simulink model "TosAeb"  (R2021a)
settings: Solver=FixedStepDiscrete  StartTime=0.0  StopTime=inf  FixedStep=0.01  RelTol=1e-3
undefined symbols (not defined in the file or in its folder): ACCModSts, AccSts1, AEB_ObjectMovingOrientation, AEB_ObjectMovingState, CN_BTCActive, CN_CurrentGearR, CN_CurrentGearR_DHT, CN_PTCActive, CN_VDCActive, CN_VSC_EspOff, CN_VSC_FCW_AEB_Error, CN_VSC_FCW_AEB_NoDispay, CN_VSC_FCW_AEB_PerfmDgdtn, CN_VSC_FCW_AEB_TempUnAvlbl, CN_VSC_VehStandstill, CNaccelAvoidMax, CNaccelAvoidMin, CNbrakePedalPress, CNcomfortStrLeftAccelMax, CNcomfortStrLeftAccelMin, CNcomfortStrRightAccelMax, CNcomfortStrRightAccelMin, CNDrvDoorOpen, CNDrvModeEco, CNDrvModeNorm, CNDrvModeOther, CNDrvModeSport, CNDrvSBRreminder, CNNull, CNobjInvalidID, CNPassDoorOpen, CNPassSBRreminder, CNPEBSAccelNegInf, CNPEBSAccelNull, CNPEBSTimeInf, CNPEBSTimeNull, CNSTOPMAX, CNSTOPNULL, CNsubjWidth, CNSwtOFF, CNSwtON, CNTTCMAX, CNTTCNULL, CNVehConfigAEBCycNone, CNVehConfigAEBNone, CNVehConfigAEBPedNone, CNVehConfigFCWNone, CNVehConfigJANone, CollisionSeverity, FALSE, FCWSnvty, JACycObjCalcInfoDefaultParams, JAPedObjCalcInfoDefaultParams, JAVehObjCalcInfoDefaultParams, latCycObjCalcInfoDefaultParams, latPedObjCalcInfoDefaultParams, longObjCalcInfoDefaultParams, ModSts, movingStatus, ObjectType ... (+1112)
libraries: simulink x3031, AEBSFunctionLib x50, Ta_Library x33, AEBSStateMachineLib x23, Mylibrary x12, simulink_extras x7, ObjSelect_lib x3, dspstat3 x2
(part 44 of 102)

== system TosAeb/FcwAebJa_Step_sys/ALG_AEBSystem/ALG_AEBController/AEB_FuncCondCalcApp/PedFunctionCondCalc/pedPrefillSuppAborCond/pedPrefillSuppCondCalc/pedPrefillObjRelActiveCondCalc ==
  ObjectID : Inport
  vxAbsObj : Inport  Port=2
  ObjFusQuality : Inport  Port=3
  dy : Inport  Port=4
  vy : Inport  Port=5
  Abs : Abs
  Compare To Constant : simulink/Logic and Bit Operations/Compare To Constant  relop=>  const=CNNull  OutDataTypeStr=boolean
  Compare To Constant1 : simulink/Logic and Bit Operations/Compare To Constant  relop=>  const=P_AEB_Func_pedPrefillVxAbsObjSuppThres  OutDataTypeStr=boolean
  Compare To Constant11 : simulink/Logic and Bit Operations/Compare To Constant  relop===  const=FALSE  OutDataTypeStr=boolean
  Compare To Constant2 : simulink/Logic and Bit Operations/Compare To Constant  relop=>  const=CNNull  OutDataTypeStr=boolean
  Compare To Constant3 : simulink/Logic and Bit Operations/Compare To Constant  relop=<  const=CNNull  OutDataTypeStr=boolean
  Compare To Constant4 : simulink/Logic and Bit Operations/Compare To Constant  relop===  const=CNobjInvalidID  OutDataTypeStr=boolean
  Compare To Constant5 : simulink/Logic and Bit Operations/Compare To Constant  relop=<  const=CNNull  OutDataTypeStr=boolean
  Logical Operator : Logic  Operator=OR  Inputs=3  AllPortsSameDT=off  OutDataTypeStr=boolean
  Logical Operator1 : Logic  AllPortsSameDT=off  OutDataTypeStr=boolean
  Logical Operator2 : Logic  AllPortsSameDT=off  OutDataTypeStr=boolean
  Logical Operator3 : Logic  Operator=OR  AllPortsSameDT=off  OutDataTypeStr=boolean
  Terminator : Terminator
  pedPrefillObjRelSuppCondFulfilled : Outport
  wires:
  Logical Operator2/1 -> Logical Operator3/2
  Logical Operator1/1 -> Logical Operator3/1
  Compare To Constant5/1 -> Logical Operator2/2
  Compare To Constant3/1 -> Logical Operator2/1
  Compare To Constant2/1 -> Logical Operator1/2
  Compare To Constant/1 -> Logical Operator1/1
  vy/1 -> Compare To Constant2/1, Compare To Constant5/1
  dy/1 -> Compare To Constant/1, Compare To Constant3/1
  Compare To Constant11/1 -> Logical Operator/3  "ObjFusQuality"
  ObjFusQuality/1 -> Compare To Constant11/1
  Logical Operator/1 -> pedPrefillObjRelSuppCondFulfilled/1
  Compare To Constant4/1 -> Logical Operator/1
  Compare To Constant1/1 -> Logical Operator/2
  Abs/1 -> Compare To Constant1/1
  vxAbsObj/1 -> Abs/1
  ObjectID/1 -> Compare To Constant4/1
  Logical Operator3/1 -> Terminator/1

== system TosAeb/FcwAebJa_Step_sys/ALG_AEBSystem/ALG_AEBController/AEB_FuncCondCalcApp/PedFunctionCondCalc/pedPrefillSuppAborCond/pedPrefillSuppCondCalc/pedPrefillSituationRelActiveCondCalc ==
  latCollisionSeverity : Inport
  subjCurvTraj : Inport  Port=2
  subjLatAccel : Inport  Port=3
  colliisonRiskProb : Inport  Port=4
  ObjectTypeCameraConfirmed : Inport  Port=5
  dy : Inport  Port=6
  vy : Inport  Port=7
  dxFrontBumper : Inport  Port=8
  longDist4Brk : Inport  Port=9
  distSecure : Inport  Port=10
  subVel : Inport  Port=11
  objLatVyCond4PrefillCalc : Subsystem
  objLongDistCond4PrefillCalc : Subsystem
  pedPrefillSituationRelSuppCond : Subsystem
  pedPrefillSituationRelSuppCondFulfilled : Outport
  wires:
  objLongDistCond4PrefillCalc/1(ObjLongDistCond4Prefill) -> pedPrefillSituationRelSuppCond/7(objLatVyCond4Prefill)
  objLatVyCond4PrefillCalc/1(ObjLatVyCond4Prefill) -> pedPrefillSituationRelSuppCond/6(objLongDistCond4Prefill)
  distSecure/1 -> objLongDistCond4PrefillCalc/3(distSecure)
  subVel/1 -> objLongDistCond4PrefillCalc/4(subVel)
  longDist4Brk/1 -> objLongDistCond4PrefillCalc/2(longDist4Brk)
  pedPrefillSituationRelSuppCond/1(pedPrefillSituationRelActiveCondFulfilled) -> pedPrefillSituationRelSuppCondFulfilled/1
  ObjectTypeCameraConfirmed/1 -> pedPrefillSituationRelSuppCond/5(ObjectTypeCameraConfirmed), objLatVyCond4PrefillCalc/1(objObjectTypeCameraConfirmed)
  colliisonRiskProb/1 -> pedPrefillSituationRelSuppCond/4(colliisonRiskProb)
  subjLatAccel/1 -> pedPrefillSituationRelSuppCond/3(subjLatAccel)
  subjCurvTraj/1 -> pedPrefillSituationRelSuppCond/2(subjCurvTraj)
  latCollisionSeverity/1 -> pedPrefillSituationRelSuppCond/1(latCollisionSeverity)
  dy/1 -> objLatVyCond4PrefillCalc/2(dy)
  vy/1 -> objLatVyCond4PrefillCalc/3(vy)
  dxFrontBumper/1 -> objLongDistCond4PrefillCalc/1(dxFrontBumper)

== system TosAeb/FcwAebJa_Step_sys/ALG_AEBSystem/ALG_AEBController/AEB_FuncCondCalcApp/PedFunctionCondCalc/pedPrefillSuppAborCond/pedPrefillSuppCondCalc/pedPrefillSuppCondFinalJudge ==
  pedPrefillObjRelSuppCondFulfilled : Inport
  pedPrefillVehRelSuppCondFulfilled : Inport  Port=2
  pedPrefillSituationRelSuppCondFulfilled : Inport  Port=3
  Compare To Constant : simulink/Logic and Bit Operations/Compare To Constant  relop===  const=TRUE  OutDataTypeStr=boolean
  Compare To Constant1 : simulink/Logic and Bit Operations/Compare To Constant  relop===  const=TRUE  OutDataTypeStr=boolean
  Compare To Constant2 : simulink/Logic and Bit Operations/Compare To Constant  relop===  const=TRUE  OutDataTypeStr=boolean
  Logical Operator : Logic  Operator=OR  Inputs=3  AllPortsSameDT=off  OutDataTypeStr=boolean
  pedPrefillSuppCondFulfilled : Outport
  wires:
  Compare To Constant2/1 -> Logical Operator/3
  Compare To Constant1/1 -> Logical Operator/2
  Compare To Constant/1 -> Logical Operator/1
  pedPrefillVehRelSuppCondFulfilled/1 -> Compare To Constant1/1
  pedPrefillObjRelSuppCondFulfilled/1 -> Compare To Constant/1
  pedPrefillSituationRelSuppCondFulfilled/1 -> Compare To Constant2/1
  Logical Operator/1 -> pedPrefillSuppCondFulfilled/1

== system TosAeb/FcwAebJa_Step_sys/ALG_AEBSystem/ALG_AEBController/AEB_FuncCondCalcApp/PedFunctionCondCalc/pedPrefillSuppAborCond/pedPrefillSuppCondCalc/pedPrefillVehRelActiveCondCalc ==
  subjVel : Inport
  VehConfigIbooster : Inport  Port=2
  ESP_MasterCylindBrakePressValid : Inport  Port=3
  ESP_MasterCylindBrakePress : Inport  Port=4
  IB_BrkPedalAppld : Inport  Port=5
  SteerWheelAngle : Inport  Port=6
  SteerWheelSpd : Inport  Port=7
  AccrPedlPosnDiagc : Inport  Port=8
  vehStateCalcpedPrefillSuppFlg : Inport  Port=9
  Abs : Abs
  Abs1 : Abs
  AccReleventSuppCondStateDelayAbortion : AEBSFunctionLib/StateDelayAbortion  SystemSampleTime=-1  VariantActivationTime=update diagram  Latency=0  AutoFrameSizeCalculation=off
  Compare To Constant : simulink/Logic and Bit Operations/Compare To Constant  relop=>  const=P_AEB_Func_pedPrefillStrWheelAngleSupThres  OutDataTypeStr=boolean
  Compare To Constant1 : simulink/Logic and Bit Operations/Compare To Constant  relop=>  const=P_AEB_Func_pedPrefillGasPedalSupThres  OutDataTypeStr=boolean
  Compare To Constant2 : simulink/Logic and Bit Operations/Compare To Constant  relop=>  const=P_AEB_Func_pedPrefillStrWheelAngleSpdSupThres  OutDataTypeStr=boolean
  Compare To Constant7 : simulink/Logic and Bit Operations/Compare To Constant  relop===  const=TRUE  OutDataTypeStr=boolean
  Compare To Constant8 : simulink/Logic and Bit Operations/Compare To Constant  relop=>  const=P_AEB_Func_pedPrefillSubjVelMax  OutDataTypeStr=boolean
  Compare To Constant9 : simulink/Logic and Bit Operations/Compare To Constant  relop=<  const=P_AEB_Func_pedPrefillSubjVelMin  OutDataTypeStr=boolean
  Constant : Constant  Value=P_AEB_Func_pedPrefillStrCondAborDelayTime
  Constant1 : Constant  Value=0.02
  Constant2 : Constant  Value=P_AEB_Func_pedPrefillAccrCondAborDelayTime
  Constant3 : Constant  Value=0.02
  Logical Operator : Logic  Operator=OR  AllPortsSameDT=off  OutDataTypeStr=boolean
  Logical Operator4 : Logic  Operator=OR  AllPortsSameDT=off  OutDataTypeStr=boolean
  Logical Operator5 : Logic  Operator=OR  Inputs=5  AllPortsSameDT=off  OutDataTypeStr=boolean
  StrReleventSuppCondDelayAbortion : AEBSFunctionLib/StateDelayAbortion  SystemSampleTime=-1  VariantActivationTime=update diagram  Latency=0  AutoFrameSizeCalculation=off
  brakeReleventSuppCond : Subsystem
  pedPrefillVehRelSuppCondFulfilled : Outport
  wires:
  VehConfigIbooster/1 -> brakeReleventSuppCond/1(VehConfigIbooster)
  IB_BrkPedalAppld/1 -> brakeReleventSuppCond/4(IB_BrkPedalAppld)
  ESP_MasterCylindBrakePress/1 -> brakeReleventSuppCond/3(ESP_MasterCylindBrakePress)
  ESP_MasterCylindBrakePressValid/1 -> brakeReleventSuppCond/2(ESP_MasterCylindBrakePressValid)
  Constant2/1 -> AccReleventSuppCondStateDelayAbortion/2
  Constant3/1 -> AccReleventSuppCondStateDelayAbortion/3
  Compare To Constant1/1 -> AccReleventSuppCondStateDelayAbortion/1
  Constant1/1 -> StrReleventSuppCondDelayAbortion/3
  Constant/1 -> StrReleventSuppCondDelayAbortion/2
  StrReleventSuppCondDelayAbortion/1 -> Logical Operator5/3  "strReleventSuppCondFulfilled"
  Logical Operator4/1 -> StrReleventSuppCondDelayAbortion/1
  AccReleventSuppCondStateDelayAbortion/1 -> Logical Operator5/4  "accReleventSuppCondFulfilled"
  Abs1/1 -> Compare To Constant2/1
  vehStateCalcpedPrefillSuppFlg/1 -> Compare To Constant7/1
  Compare To Constant8/1 -> Logical Operator/1
  Compare To Constant2/1 -> Logical Operator4/2
  SteerWheelSpd/1 -> Abs1/1
  Compare To Constant/1 -> Logical Operator4/1
  Abs/1 -> Compare To Constant/1
  SteerWheelAngle/1 -> Abs/1
  brakeReleventSuppCond/1(brakeReleventSuppCond) -> Logical Operator5/2  "BrkReleventSuppCondFulfilled"
  Compare To Constant9/1 -> Logical Operator/2
  AccrPedlPosnDiagc/1 -> Compare To Constant1/1
  Compare To Constant7/1 -> Logical Operator5/5  "vehStateCalcPedPrefillSuppCondFulfilled"
  Logical Operator/1 -> Logical Operator5/1  "subjVelReleventSuppCondFulfilled"
  subjVel/1 -> Compare To Constant9/1, Compare To Constant8/1
  Logical Operator5/1 -> pedPrefillVehRelSuppCondFulfilled/1  "pedPrefillVehRelSuppCondFulfilled"

== system TosAeb/FcwAebJa_Step_sys/ALG_AEBSystem/ALG_AEBController/AEB_FuncImpManagementApp/FuncImpArbCalc/BrkFuncImpArbCalc/BrkFuncImpAborCondCalc/AEBfullFuncImpAborCondCalc ==
  pedAEBfullModStsArb : Inport
  cycAEBfullModStsArb : Inport  Port=2
  EBAModStsArb : Inport  Port=3
  JA_PTW_AEBfullModStsArb : Inport  Port=4
  JA_cycAEBfullModStsArb : Inport  Port=5
  JA_pedAEBfullModStsArb : Inport  Port=6
  Compare To Constant{3..5,23..25} (6 alike) : simulink/Logic and Bit Operations/Compare To Constant  relop===  const=ModSts.ActiveState  OutDataTypeStr=boolean
  Logical Operator4 : Logic  Operator=OR  Inputs=6  AllPortsSameDT=off  OutDataTypeStr=boolean
  AEBfullFuncImpAborCond : Outport
  wires:
  Compare To Constant3/1 -> Logical Operator4/4
  Compare To Constant4/1 -> Logical Operator4/5
  Compare To Constant5/1 -> Logical Operator4/6
  JA_pedAEBfullModStsArb/1 -> Compare To Constant5/1
  JA_cycAEBfullModStsArb/1 -> Compare To Constant4/1
  JA_PTW_AEBfullModStsArb/1 -> Compare To Constant3/1
  EBAModStsArb/1 -> Compare To Constant25/1
  Compare To Constant24/1 -> Logical Operator4/2
  Compare To Constant23/1 -> Logical Operator4/1
  cycAEBfullModStsArb/1 -> Compare To Constant24/1
  pedAEBfullModStsArb/1 -> Compare To Constant23/1
  Logical Operator4/1 -> AEBfullFuncImpAborCond/1
  Compare To Constant25/1 -> Logical Operator4/3

== system TosAeb/FcwAebJa_Step_sys/ALG_AEBSystem/ALG_AEBController/AEB_FuncImpManagementApp/FuncImpArbCalc/BrkFuncImpArbCalc/BrkFuncImpAborCondCalc/AEBpartFuncImpAborCondCalc ==
  AEBfullModStsArb : Inport
  pedAEBfullModStsArb : Inport  Port=2
  cycAEBpartModStsArb : Inport  Port=3
  cycAEBfullModStsArb : Inport  Port=4
  JA_vehAEBfullModStsArb : Inport  Port=5
  EBAModStsArb : Inport  Port=6
  JA_PTW_AEBfullModStsArb : Inport  Port=7
  JA_cycAEBfullModStsArb : Inport  Port=8
  JA_pedAEBfullModStsArb : Inport  Port=9
  Compare To Constant{1..3,7..12} (9 alike) : simulink/Logic and Bit Operations/Compare To Constant  relop===  const=ModSts.ActiveState  OutDataTypeStr=boolean
  Logical Operator1 : Logic  Operator=OR  Inputs=9  AllPortsSameDT=off  OutDataTypeStr=boolean
  AEBpartFuncImpAborCond : Outport
  wires:
  Compare To Constant3/1 -> Logical Operator1/9
  Compare To Constant2/1 -> Logical Operator1/8
  Compare To Constant1/1 -> Logical Operator1/7
  JA_pedAEBfullModStsArb/1 -> Compare To Constant3/1
  JA_cycAEBfullModStsArb/1 -> Compare To Constant2/1
  JA_PTW_AEBfullModStsArb/1 -> Compare To Constant1/1
  Logical Operator1/1 -> AEBpartFuncImpAborCond/1
  Compare To Constant9/1 -> Logical Operator1/3
  Compare To Constant11/1 -> Logical Operator1/5
  pedAEBfullModStsArb/1 -> Compare To Constant8/1
  Compare To Constant10/1 -> Logical Operator1/4
  Compare To Constant7/1 -> Logical Operator1/1
  Compare To Constant8/1 -> Logical Operator1/2
  EBAModStsArb/1 -> Compare To Constant12/1
  AEBfullModStsArb/1 -> Compare To Constant7/1
  cycAEBpartModStsArb/1 -> Compare To Constant9/1
  cycAEBfullModStsArb/1 -> Compare To Constant10/1
  JA_vehAEBfullModStsArb/1 -> Compare To Constant11/1
  Compare To Constant12/1 -> Logical Operator1/6

== system TosAeb/FcwAebJa_Step_sys/ALG_AEBSystem/ALG_AEBController/AEB_FuncImpManagementApp/FuncImpArbCalc/BrkFuncImpArbCalc/BrkFuncImpAborCondCalc/EBAFuncImpAborCondCalc ==
  Constant : Constant  Value=FALSE
  EBAFuncImpAborCond : Outport
  wires:
  Constant/1 -> EBAFuncImpAborCond/1

== system TosAeb/FcwAebJa_Step_sys/ALG_AEBSystem/ALG_AEBController/AEB_FuncImpManagementApp/FuncImpArbCalc/BrkFuncImpArbCalc/BrkFuncImpAborCondCalc/FCTBFuncImpAborCondCalc ==
  AEBpartModStsArb : Inport
  AEBfullModStsArb : Inport  Port=2
  EBAModStsArb : Inport  Port=3
  pedAEBfullModStsArb : Inport  Port=4
  cycAEBfullModStsArb : Inport  Port=5
  cycAEBpartModStsArb : Inport  Port=6
  JA_vehAEBfullModStsArb : Inport  Port=7
  JA_PTW_AEBModStsArb : Inport  Port=8
  JA_cycAEBModStsArb : Inport  Port=9
  JA_pedAEBModStsArb : Inport  Port=10
  Compare To Constant{,1..9} (10 alike) : simulink/Logic and Bit Operations/Compare To Constant  relop===  const=ModSts.ActiveState  OutDataTypeStr=boolean
  Logical Operator : Logic  Operator=OR  Inputs=10  AllPortsSameDT=off  OutDataTypeStr=boolean
  FCTBFuncImpAborCond : Outport
  wires:
  JA_pedAEBModStsArb/1 -> Compare To Constant9/1
  Compare To Constant9/1 -> Logical Operator/10
  JA_cycAEBModStsArb/1 -> Compare To Constant8/1
  Compare To Constant8/1 -> Logical Operator/9
  Compare To Constant7/1 -> Logical Operator/8
  JA_PTW_AEBModStsArb/1 -> Compare To Constant7/1
  Compare To Constant2/1 -> Logical Operator/3
  Compare To Constant5/1 -> Logical Operator/6
  Compare To Constant/1 -> Logical Operator/1
  Compare To Constant4/1 -> Logical Operator/5
  EBAModStsArb/1 -> Compare To Constant2/1
  Compare To Constant6/1 -> Logical Operator/7
  Compare To Constant3/1 -> Logical Operator/4
  Logical Operator/1 -> FCTBFuncImpAborCond/1
  Compare To Constant1/1 -> Logical Operator/2
  pedAEBfullModStsArb/1 -> Compare To Constant3/1
  cycAEBfullModStsArb/1 -> Compare To Constant4/1
  AEBfullModStsArb/1 -> Compare To Constant1/1
  AEBpartModStsArb/1 -> Compare To Constant/1
  JA_vehAEBfullModStsArb/1 -> Compare To Constant6/1
  cycAEBpartModStsArb/1 -> Compare To Constant5/1

== system TosAeb/FcwAebJa_Step_sys/ALG_AEBSystem/ALG_AEBController/AEB_FuncImpManagementApp/FuncImpArbCalc/BrkFuncImpArbCalc/BrkFuncImpAborCondCalc/JA_PTW_AEBfullFuncImpAborCondCalc ==
  AEBfullModStsArb : Inport
  pedAEBfullModStsArb : Inport  Port=2
  JA_pedAEBfullModStsArb : Inport  Port=3
  cycAEBfullModStsArb : Inport  Port=4
  EBAModStsArb : Inport  Port=5
  Compare To Constant{7..10,12} (5 alike) : simulink/Logic and Bit Operations/Compare To Constant  relop===  const=ModSts.ActiveState  OutDataTypeStr=boolean
  Logical Operator1 : Logic  Operator=OR  Inputs=5  AllPortsSameDT=off  OutDataTypeStr=boolean
  JA_PTW_AEBfullFuncImpAborCond : Outport
  wires:
  Compare To Constant12/1 -> Logical Operator1/5
  cycAEBfullModStsArb/1 -> Compare To Constant10/1
  JA_pedAEBfullModStsArb/1 -> Compare To Constant9/1
  AEBfullModStsArb/1 -> Compare To Constant7/1
  EBAModStsArb/1 -> Compare To Constant12/1
  Compare To Constant8/1 -> Logical Operator1/2
  Compare To Constant7/1 -> Logical Operator1/1
  Compare To Constant10/1 -> Logical Operator1/4
  pedAEBfullModStsArb/1 -> Compare To Constant8/1
  Compare To Constant9/1 -> Logical Operator1/3
  Logical Operator1/1 -> JA_PTW_AEBfullFuncImpAborCond/1

== system TosAeb/FcwAebJa_Step_sys/ALG_AEBSystem/ALG_AEBController/AEB_FuncImpManagementApp/FuncImpArbCalc/BrkFuncImpArbCalc/BrkFuncImpAborCondCalc/JA_cycAEBfullFuncImpAborCondCalc ==
  cycAEBfullModStsArb : Inport
  pedAEBfullModStsArb : Inport  Port=2
  EBAModStsArb : Inport  Port=3
  JA_pedAEBModStsArb : Inport  Port=4
  Compare To Constant{1,19..21} (4 alike) : simulink/Logic and Bit Operations/Compare To Constant  relop===  const=ModSts.ActiveState  OutDataTypeStr=boolean
  Logical Operator3 : Logic  Operator=OR  Inputs=4  AllPortsSameDT=off  OutDataTypeStr=boolean
  JA_cycAEBfullFuncImpAborCond : Outport
  wires:
  Compare To Constant1/1 -> Logical Operator3/4
  JA_pedAEBModStsArb/1 -> Compare To Constant1/1
  Compare To Constant19/1 -> Logical Operator3/1
  Compare To Constant21/1 -> Logical Operator3/3
  Compare To Constant20/1 -> Logical Operator3/2
  pedAEBfullModStsArb/1 -> Compare To Constant20/1
  EBAModStsArb/1 -> Compare To Constant21/1
  Logical Operator3/1 -> JA_cycAEBfullFuncImpAborCond/1
  cycAEBfullModStsArb/1 -> Compare To Constant19/1

== system TosAeb/FcwAebJa_Step_sys/ALG_AEBSystem/ALG_AEBController/AEB_FuncImpManagementApp/FuncImpArbCalc/BrkFuncImpArbCalc/BrkFuncImpAborCondCalc/JA_pedAEBfullFuncImpAborCondCalc ==
  cycAEBfullModStsArb : Inport
  pedAEBfullModStsArb : Inport  Port=2
  EBAModStsArb : Inport  Port=3
  Compare To Constant19 : simulink/Logic and Bit Operations/Compare To Constant  relop===  const=ModSts.ActiveState  OutDataTypeStr=boolean
  Compare To Constant20 : simulink/Logic and Bit Operations/Compare To Constant  relop===  const=ModSts.ActiveState  OutDataTypeStr=boolean
  Compare To Constant21 : simulink/Logic and Bit Operations/Compare To Constant  relop===  const=ModSts.ActiveState  OutDataTypeStr=boolean
  Logical Operator3 : Logic  Operator=OR  Inputs=3  AllPortsSameDT=off  OutDataTypeStr=boolean
  JA_pedAEBfullFuncImpAborCond : Outport
  wires:
  cycAEBfullModStsArb/1 -> Compare To Constant19/1
  Logical Operator3/1 -> JA_pedAEBfullFuncImpAborCond/1
  EBAModStsArb/1 -> Compare To Constant21/1
  pedAEBfullModStsArb/1 -> Compare To Constant20/1
  Compare To Constant20/1 -> Logical Operator3/2
  Compare To Constant21/1 -> Logical Operator3/3
  Compare To Constant19/1 -> Logical Operator3/1

== system TosAeb/FcwAebJa_Step_sys/ALG_AEBSystem/ALG_AEBController/AEB_FuncImpManagementApp/FuncImpArbCalc/BrkFuncImpArbCalc/BrkFuncImpAborCondCalc/JA_vehAEBfullFuncImpAborCondCalc ==
  AEBfullModStsArb : Inport
  cycAEBfullModStsArb : Inport  Port=2
  pedAEBfullModStsArb : Inport  Port=3
  EBAModStsArb : Inport  Port=4
  JA_PTW_AEBModStsArb : Inport  Port=5
  JA_cycAEBModStsArb : Inport  Port=6
  JA_pedAEBModStsArb : Inport  Port=7
  Compare To Constant{1..3,18..21} (7 alike) : simulink/Logic and Bit Operations/Compare To Constant  relop===  const=ModSts.ActiveState  OutDataTypeStr=boolean
  Logical Operator3 : Logic  Operator=OR  Inputs=7  AllPortsSameDT=off  OutDataTypeStr=boolean
  JA_vehAEBfullFuncImpAborCond : Outport
  wires:
  Compare To Constant3/1 -> Logical Operator3/7
  Compare To Constant2/1 -> Logical Operator3/6
  Compare To Constant1/1 -> Logical Operator3/5
  JA_pedAEBModStsArb/1 -> Compare To Constant3/1
  JA_cycAEBModStsArb/1 -> Compare To Constant2/1
  JA_PTW_AEBModStsArb/1 -> Compare To Constant1/1
  Compare To Constant19/1 -> Logical Operator3/2
  Compare To Constant21/1 -> Logical Operator3/4
  Compare To Constant20/1 -> Logical Operator3/3
  AEBfullModStsArb/1 -> Compare To Constant18/1
  pedAEBfullModStsArb/1 -> Compare To Constant20/1
  EBAModStsArb/1 -> Compare To Constant21/1
  Logical Operator3/1 -> JA_vehAEBfullFuncImpAborCond/1
  Compare To Constant18/1 -> Logical Operator3/1
  cycAEBfullModStsArb/1 -> Compare To Constant19/1

== system TosAeb/FcwAebJa_Step_sys/ALG_AEBSystem/ALG_AEBController/AEB_FuncImpManagementApp/FuncImpArbCalc/BrkFuncImpArbCalc/BrkFuncImpAborCondCalc/cycAEBfullFuncImpAborCondCalc ==
  pedAEBfullModStsArb : Inport
  EBAModStsArb : Inport  Port=2
  Compare To Constant22 : simulink/Logic and Bit Operations/Compare To Constant  relop===  const=ModSts.ActiveState  OutDataTypeStr=boolean
  Compare To Constant26 : simulink/Logic and Bit Operations/Compare To Constant  relop===  const=ModSts.ActiveState  OutDataTypeStr=boolean
  Logical Operator5 : Logic  Operator=OR  AllPortsSameDT=off  OutDataTypeStr=boolean
  cycAEBfullFuncImpAborCond : Outport
  wires:
  EBAModStsArb/1 -> Compare To Constant26/1
  Compare To Constant26/1 -> Logical Operator5/2
  Compare To Constant22/1 -> Logical Operator5/1
  pedAEBfullModStsArb/1 -> Compare To Constant22/1
  Logical Operator5/1 -> cycAEBfullFuncImpAborCond/1

== system TosAeb/FcwAebJa_Step_sys/ALG_AEBSystem/ALG_AEBController/AEB_FuncImpManagementApp/FuncImpArbCalc/BrkFuncImpArbCalc/BrkFuncImpAborCondCalc/cycAEBpartFuncImpAborCondCalc ==
  AEBfullModStsArb : Inport
  pedAEBfullModStsArb : Inport  Port=2
  cycAEBfullModStsArb : Inport  Port=3
  JA_vehAEBfullModStsArb : Inport  Port=4
  EBAModStsArb : Inport  Port=5
  JA_PTW_AEBfullModStsArb : Inport  Port=6
  JA_cycAEBfullModStsArb : Inport  Port=7
  JA_pedAEBfullModStsArb : Inport  Port=8
  Compare To Constant{1..3,13..17} (8 alike) : simulink/Logic and Bit Operations/Compare To Constant  relop===  const=ModSts.ActiveState  OutDataTypeStr=boolean
  Logical Operator2 : Logic  Operator=OR  Inputs=8  AllPortsSameDT=off  OutDataTypeStr=boolean
  cycAEBpartFuncImpAborCond : Outport
  wires:
  Compare To Constant3/1 -> Logical Operator2/8
  Compare To Constant2/1 -> Logical Operator2/7
  Compare To Constant1/1 -> Logical Operator2/6
  JA_pedAEBfullModStsArb/1 -> Compare To Constant3/1
  JA_cycAEBfullModStsArb/1 -> Compare To Constant2/1
  JA_PTW_AEBfullModStsArb/1 -> Compare To Constant1/1
  pedAEBfullModStsArb/1 -> Compare To Constant14/1
  Compare To Constant17/1 -> Logical Operator2/5
  Compare To Constant13/1 -> Logical Operator2/1
  Compare To Constant15/1 -> Logical Operator2/3
  Compare To Constant16/1 -> Logical Operator2/4
  JA_vehAEBfullModStsArb/1 -> Compare To Constant16/1
  Compare To Constant14/1 -> Logical Operator2/2
  Logical Operator2/1 -> cycAEBpartFuncImpAborCond/1
  EBAModStsArb/1 -> Compare To Constant17/1
  AEBfullModStsArb/1 -> Compare To Constant13/1
  cycAEBfullModStsArb/1 -> Compare To Constant15/1

== system TosAeb/FcwAebJa_Step_sys/ALG_AEBSystem/ALG_AEBController/AEB_FuncImpManagementApp/FuncImpArbCalc/BrkFuncImpArbCalc/BrkFuncImpAborCondCalc/pedAEBfullFuncImpAborCondCalc ==
  EBAModStsArb : Inport
  Compare To Constant27 : simulink/Logic and Bit Operations/Compare To Constant  relop===  const=ModSts.ActiveState  OutDataTypeStr=boolean
  pedAEBfullFuncImpAborCond : Outport
  wires:
  EBAModStsArb/1 -> Compare To Constant27/1
  Compare To Constant27/1 -> pedAEBfullFuncImpAborCond/1

== system TosAeb/FcwAebJa_Step_sys/ALG_AEBSystem/ALG_AEBController/AEB_FuncImpManagementApp/FuncImpArbCalc/BrkFuncImpArbCalc/BrkFuncImpAborCondCalc1/AEBfullFuncImpAborCondCalc ==
  pedAEBfullModStsArb : Inport
  cycAEBfullModStsArb : Inport  Port=2
  EBAModStsArb : Inport  Port=3
  Compare To Constant23 : simulink/Logic and Bit Operations/Compare To Constant  relop===  const=ModSts.ActiveState  OutDataTypeStr=boolean
  Compare To Constant24 : simulink/Logic and Bit Operations/Compare To Constant  relop===  const=ModSts.ActiveState  OutDataTypeStr=boolean
  Compare To Constant25 : simulink/Logic and Bit Operations/Compare To Constant  relop===  const=ModSts.ActiveState  OutDataTypeStr=boolean
  Logical Operator4 : Logic  Operator=OR  Inputs=3  AllPortsSameDT=off  OutDataTypeStr=boolean
  AEBfullFuncImpAborCond : Outport
  wires:
  Compare To Constant25/1 -> Logical Operator4/3
  Logical Operator4/1 -> AEBfullFuncImpAborCond/1
  pedAEBfullModStsArb/1 -> Compare To Constant23/1
  cycAEBfullModStsArb/1 -> Compare To Constant24/1
  Compare To Constant23/1 -> Logical Operator4/1
  Compare To Constant24/1 -> Logical Operator4/2
  EBAModStsArb/1 -> Compare To Constant25/1

== system TosAeb/FcwAebJa_Step_sys/ALG_AEBSystem/ALG_AEBController/AEB_FuncImpManagementApp/FuncImpArbCalc/BrkFuncImpArbCalc/BrkFuncImpAborCondCalc1/AEBpartFuncImpAborCondCalc ==
  AEBfullModStsArb : Inport
  pedAEBfullModStsArb : Inport  Port=2
  JA_pedAEBfullModStsArb : Inport  Port=3
  cycAEBpartModStsArb : Inport  Port=4
  cycAEBfullModStsArb : Inport  Port=5
  JA_vehAEBfullModStsArb : Inport  Port=6
  EBAModStsArb : Inport  Port=7
  Compare To Constant{1,7..12} (7 alike) : simulink/Logic and Bit Operations/Compare To Constant  relop===  const=ModSts.ActiveState  OutDataTypeStr=boolean
  Logical Operator1 : Logic  Operator=OR  Inputs=7  AllPortsSameDT=off  OutDataTypeStr=boolean
  AEBpartFuncImpAborCond : Outport
  wires:
  Compare To Constant12/1 -> Logical Operator1/7
  JA_vehAEBfullModStsArb/1 -> Compare To Constant11/1
  cycAEBfullModStsArb/1 -> Compare To Constant10/1
  cycAEBpartModStsArb/1 -> Compare To Constant9/1
  AEBfullModStsArb/1 -> Compare To Constant7/1
  EBAModStsArb/1 -> Compare To Constant12/1
  Compare To Constant8/1 -> Logical Operator1/2
  Compare To Constant7/1 -> Logical Operator1/1
  Compare To Constant10/1 -> Logical Operator1/5
  pedAEBfullModStsArb/1 -> Compare To Constant8/1
  Compare To Constant11/1 -> Logical Operator1/6
  Compare To Constant9/1 -> Logical Operator1/4
  Logical Operator1/1 -> AEBpartFuncImpAborCond/1
  JA_pedAEBfullModStsArb/1 -> Compare To Constant1/1
  Compare To Constant1/1 -> Logical Operator1/3

== system TosAeb/FcwAebJa_Step_sys/ALG_AEBSystem/ALG_AEBController/AEB_FuncImpManagementApp/FuncImpArbCalc/BrkFuncImpArbCalc/BrkFuncImpAborCondCalc1/EBAFuncImpAborCondCalc ==
  Constant : Constant  Value=FALSE
  EBAFuncImpAborCond : Outport
  wires:
  Constant/1 -> EBAFuncImpAborCond/1

== system TosAeb/FcwAebJa_Step_sys/ALG_AEBSystem/ALG_AEBController/AEB_FuncImpManagementApp/FuncImpArbCalc/BrkFuncImpArbCalc/BrkFuncImpAborCondCalc1/FCTBFuncImpAborCondCalc ==
  AEBpartModStsArb : Inport
  AEBfullModStsArb : Inport  Port=2
  EBAModStsArb : Inport  Port=3
  pedAEBfullModStsArb : Inport  Port=4
  cycAEBfullModStsArb : Inport  Port=5
  cycAEBpartModStsArb : Inport  Port=6
  JA_vehAEBfullModStsArb : Inport  Port=7
  JA_PTW_AEBModStsArb : Inport  Port=8
  JA_cycAEBModStsArb : Inport  Port=9
  JA_pedAEBModStsArb : Inport  Port=10
  Compare To Constant{,1..9} (10 alike) : simulink/Logic and Bit Operations/Compare To Constant  relop===  const=ModSts.ActiveState  OutDataTypeStr=boolean
  Logical Operator : Logic  Operator=OR  Inputs=10  AllPortsSameDT=off  OutDataTypeStr=boolean
  FCTBFuncImpAborCond : Outport
  wires:
  cycAEBpartModStsArb/1 -> Compare To Constant5/1
  JA_vehAEBfullModStsArb/1 -> Compare To Constant6/1
  AEBpartModStsArb/1 -> Compare To Constant/1
  AEBfullModStsArb/1 -> Compare To Constant1/1
  cycAEBfullModStsArb/1 -> Compare To Constant4/1
  pedAEBfullModStsArb/1 -> Compare To Constant3/1
  Compare To Constant1/1 -> Logical Operator/2
  Logical Operator/1 -> FCTBFuncImpAborCond/1
  Compare To Constant3/1 -> Logical Operator/4
  Compare To Constant6/1 -> Logical Operator/7
  EBAModStsArb/1 -> Compare To Constant2/1
  Compare To Constant4/1 -> Logical Operator/5
  Compare To Constant/1 -> Logical Operator/1
  Compare To Constant5/1 -> Logical Operator/6
  Compare To Constant2/1 -> Logical Operator/3
  JA_PTW_AEBModStsArb/1 -> Compare To Constant7/1
  Compare To Constant7/1 -> Logical Operator/8
  Compare To Constant8/1 -> Logical Operator/9
  JA_cycAEBModStsArb/1 -> Compare To Constant8/1
  Compare To Constant9/1 -> Logical Operator/10
  JA_pedAEBModStsArb/1 -> Compare To Constant9/1

== system TosAeb/FcwAebJa_Step_sys/ALG_AEBSystem/ALG_AEBController/AEB_FuncImpManagementApp/FuncImpArbCalc/BrkFuncImpArbCalc/BrkFuncImpAborCondCalc1/JA_PTW_AEBfullFuncImpAborCondCalc ==
  AEBfullModStsArb : Inport
  pedAEBfullModStsArb : Inport  Port=2
  cycJA_PTW_AEBfullModStsArb : Inport  Port=3
  cycAEBfullModStsArb : Inport  Port=4
  JA_vehAEBfullModStsArb : Inport  Port=5
  EBAModStsArb : Inport  Port=6
  Compare To Constant{7..12} (6 alike) : simulink/Logic and Bit Operations/Compare To Constant  relop===  const=ModSts.ActiveState  OutDataTypeStr=boolean
  Logical Operator1 : Logic  Operator=OR  Inputs=6  AllPortsSameDT=off  OutDataTypeStr=boolean
  JA_PTW_AEBfullFuncImpAborCond : Outport
  wires:
  Logical Operator1/1 -> JA_PTW_AEBfullFuncImpAborCond/1
  Compare To Constant9/1 -> Logical Operator1/3
  Compare To Constant11/1 -> Logical Operator1/5
  pedAEBfullModStsArb/1 -> Compare To Constant8/1
  Compare To Constant10/1 -> Logical Operator1/4
  Compare To Constant7/1 -> Logical Operator1/1
  Compare To Constant8/1 -> Logical Operator1/2
  EBAModStsArb/1 -> Compare To Constant12/1
  AEBfullModStsArb/1 -> Compare To Constant7/1
  cycJA_PTW_AEBfullModStsArb/1 -> Compare To Constant9/1
  cycAEBfullModStsArb/1 -> Compare To Constant10/1
  JA_vehAEBfullModStsArb/1 -> Compare To Constant11/1
  Compare To Constant12/1 -> Logical Operator1/6

== system TosAeb/FcwAebJa_Step_sys/ALG_AEBSystem/ALG_AEBController/AEB_FuncImpManagementApp/FuncImpArbCalc/BrkFuncImpArbCalc/BrkFuncImpAborCondCalc1/JA_cycAEBfullFuncImpAborCondCalc ==
  AEBfullModStsArb : Inport
  cycAEBfullModStsArb : Inport  Port=2
  pedAEBfullModStsArb : Inport  Port=3
  EBAModStsArb : Inport  Port=4
  JA_pedAEBModStsArb : Inport  Port=5
  Compare To Constant{1,18..21} (5 alike) : simulink/Logic and Bit Operations/Compare To Constant  relop===  const=ModSts.ActiveState  OutDataTypeStr=boolean
  Logical Operator3 : Logic  Operator=OR  Inputs=5  AllPortsSameDT=off  OutDataTypeStr=boolean
  JA_cycAEBfullFuncImpAborCond : Outport
  wires:
  cycAEBfullModStsArb/1 -> Compare To Constant19/1
  Compare To Constant18/1 -> Logical Operator3/1
  Logical Operator3/1 -> JA_cycAEBfullFuncImpAborCond/1
  EBAModStsArb/1 -> Compare To Constant21/1
  pedAEBfullModStsArb/1 -> Compare To Constant20/1
  AEBfullModStsArb/1 -> Compare To Constant18/1
  Compare To Constant20/1 -> Logical Operator3/3
  Compare To Constant21/1 -> Logical Operator3/4
  Compare To Constant19/1 -> Logical Operator3/2
  JA_pedAEBModStsArb/1 -> Compare To Constant1/1
  Compare To Constant1/1 -> Logical Operator3/5

== system TosAeb/FcwAebJa_Step_sys/ALG_AEBSystem/ALG_AEBController/AEB_FuncImpManagementApp/FuncImpArbCalc/BrkFuncImpArbCalc/BrkFuncImpAborCondCalc1/JA_pedAEBfullFuncImpAborCondCalc ==
  AEBfullModStsArb : Inport
  cycAEBfullModStsArb : Inport  Port=2
  pedAEBfullModStsArb : Inport  Port=3
  EBAModStsArb : Inport  Port=4
  Compare To Constant{18..21} (4 alike) : simulink/Logic and Bit Operations/Compare To Constant  relop===  const=ModSts.ActiveState  OutDataTypeStr=boolean
  Logical Operator3 : Logic  Operator=OR  Inputs=4  AllPortsSameDT=off  OutDataTypeStr=boolean
  JA_pedAEBfullFuncImpAborCond : Outport
  wires:
  Compare To Constant19/1 -> Logical Operator3/2
  Compare To Constant21/1 -> Logical Operator3/4
  Compare To Constant20/1 -> Logical Operator3/3
  AEBfullModStsArb/1 -> Compare To Constant18/1
  pedAEBfullModStsArb/1 -> Compare To Constant20/1
  EBAModStsArb/1 -> Compare To Constant21/1
  Logical Operator3/1 -> JA_pedAEBfullFuncImpAborCond/1
  Compare To Constant18/1 -> Logical Operator3/1
  cycAEBfullModStsArb/1 -> Compare To Constant19/1

== system TosAeb/FcwAebJa_Step_sys/ALG_AEBSystem/ALG_AEBController/AEB_FuncImpManagementApp/FuncImpArbCalc/BrkFuncImpArbCalc/BrkFuncImpAborCondCalc1/JA_vehAEBfullFuncImpAborCondCalc ==
  AEBfullModStsArb : Inport
  cycAEBfullModStsArb : Inport  Port=2
  pedAEBfullModStsArb : Inport  Port=3
  EBAModStsArb : Inport  Port=4
  JA_PTW_AEBModStsArb : Inport  Port=5
  JA_cycAEBModStsArb : Inport  Port=6
  JA_pedAEBModStsArb : Inport  Port=7
  Compare To Constant{1..3,18..21} (7 alike) : simulink/Logic and Bit Operations/Compare To Constant  relop===  const=ModSts.ActiveState  OutDataTypeStr=boolean
  Logical Operator3 : Logic  Operator=OR  Inputs=7  AllPortsSameDT=off  OutDataTypeStr=boolean
  JA_vehAEBfullFuncImpAborCond : Outport
  wires:
  cycAEBfullModStsArb/1 -> Compare To Constant19/1
  Compare To Constant18/1 -> Logical Operator3/1
  Logical Operator3/1 -> JA_vehAEBfullFuncImpAborCond/1
  EBAModStsArb/1 -> Compare To Constant21/1
  pedAEBfullModStsArb/1 -> Compare To Constant20/1
  AEBfullModStsArb/1 -> Compare To Constant18/1
  Compare To Constant20/1 -> Logical Operator3/3
  Compare To Constant21/1 -> Logical Operator3/4
  Compare To Constant19/1 -> Logical Operator3/2
  JA_PTW_AEBModStsArb/1 -> Compare To Constant1/1
  JA_cycAEBModStsArb/1 -> Compare To Constant2/1
  JA_pedAEBModStsArb/1 -> Compare To Constant3/1
  Compare To Constant1/1 -> Logical Operator3/5
  Compare To Constant2/1 -> Logical Operator3/6
  Compare To Constant3/1 -> Logical Operator3/7

== system TosAeb/FcwAebJa_Step_sys/ALG_AEBSystem/ALG_AEBController/AEB_FuncImpManagementApp/FuncImpArbCalc/BrkFuncImpArbCalc/BrkFuncImpAborCondCalc1/cycAEBfullFuncImpAborCondCalc ==
  pedAEBfullModStsArb : Inport
  EBAModStsArb : Inport  Port=2
  Compare To Constant22 : simulink/Logic and Bit Operations/Compare To Constant  relop===  const=ModSts.ActiveState  OutDataTypeStr=boolean
  Compare To Constant26 : simulink/Logic and Bit Operations/Compare To Constant  relop===  const=ModSts.ActiveState  OutDataTypeStr=boolean
  Logical Operator5 : Logic  Operator=OR  AllPortsSameDT=off  OutDataTypeStr=boolean
  cycAEBfullFuncImpAborCond : Outport
  wires:
  Logical Operator5/1 -> cycAEBfullFuncImpAborCond/1
  pedAEBfullModStsArb/1 -> Compare To Constant22/1
  Compare To Constant22/1 -> Logical Operator5/1
  Compare To Constant26/1 -> Logical Operator5/2
  EBAModStsArb/1 -> Compare To Constant26/1

== system TosAeb/FcwAebJa_Step_sys/ALG_AEBSystem/ALG_AEBController/AEB_FuncImpManagementApp/FuncImpArbCalc/BrkFuncImpArbCalc/BrkFuncImpAborCondCalc1/cycAEBpartFuncImpAborCondCalc ==
  AEBfullModStsArb : Inport
  pedAEBfullModStsArb : Inport  Port=2
  JA_pedAEBfullModStsArb : Inport  Port=3
  cycAEBfullModStsArb : Inport  Port=4
  JA_vehAEBfullModStsArb : Inport  Port=5
  EBAModStsArb : Inport  Port=6
  Compare To Constant{1,13..17} (6 alike) : simulink/Logic and Bit Operations/Compare To Constant  relop===  const=ModSts.ActiveState  OutDataTypeStr=boolean
  Logical Operator2 : Logic  Operator=OR  Inputs=6  AllPortsSameDT=off  OutDataTypeStr=boolean
  cycAEBpartFuncImpAborCond : Outport
  wires:
  cycAEBfullModStsArb/1 -> Compare To Constant15/1
  AEBfullModStsArb/1 -> Compare To Constant13/1
  EBAModStsArb/1 -> Compare To Constant17/1
  Logical Operator2/1 -> cycAEBpartFuncImpAborCond/1
  Compare To Constant14/1 -> Logical Operator2/2
  JA_vehAEBfullModStsArb/1 -> Compare To Constant16/1
  Compare To Constant16/1 -> Logical Operator2/5
  Compare To Constant15/1 -> Logical Operator2/4
  Compare To Constant13/1 -> Logical Operator2/1
  Compare To Constant17/1 -> Logical Operator2/6
  pedAEBfullModStsArb/1 -> Compare To Constant14/1
  JA_pedAEBfullModStsArb/1 -> Compare To Constant1/1
  Compare To Constant1/1 -> Logical Operator2/3

== system TosAeb/FcwAebJa_Step_sys/ALG_AEBSystem/ALG_AEBController/AEB_FuncImpManagementApp/FuncImpArbCalc/BrkFuncImpArbCalc/BrkFuncImpAborCondCalc1/pedAEBfullFuncImpAborCondCalc ==
  EBAModStsArb : Inport
  Compare To Constant27 : simulink/Logic and Bit Operations/Compare To Constant  relop===  const=ModSts.ActiveState  OutDataTypeStr=boolean
  pedAEBfullFuncImpAborCond : Outport
  wires:
  Compare To Constant27/1 -> pedAEBfullFuncImpAborCond/1
  EBAModStsArb/1 -> Compare To Constant27/1

== system TosAeb/FcwAebJa_Step_sys/ALG_AEBSystem/ALG_AEBController/AEB_FuncImpManagementApp/FuncImpArbCalc/BrkFuncImpArbCalc/BrkFuncImpArbCalc/AEBfullFuncImpArbCalc ==
  AEBfullModStsArb : Inport
  AEBfullFuncImpAborCond : Inport  Port=2
  Compare To Constant : simulink/Logic and Bit Operations/Compare To Constant  relop=~=  const=ModSts.ActiveState  OutDataTypeStr=boolean
  Compare To Constant1 : simulink/Logic and Bit Operations/Compare To Constant  relop===  const=FALSE  OutDataTypeStr=boolean
  Constant : Constant  Value=ModSts.StandbyState
  Switch : Switch  Criteria=u2 ~= 0
  Switch1 : Switch  Criteria=u2 ~= 0
  AEBfullFuncImpArb : Outport
  wires:
  Switch/1 -> AEBfullFuncImpArb/1
  Switch1/1 -> Switch/3
  Constant/1 -> Switch1/3
  Compare To Constant1/1 -> Switch1/2
  AEBfullFuncImpAborCond/1 -> Compare To Constant1/1
  Compare To Constant/1 -> Switch/2
  AEBfullModStsArb/1 -> Compare To Constant/1, Switch/1, Switch1/1

== system TosAeb/FcwAebJa_Step_sys/ALG_AEBSystem/ALG_AEBController/AEB_FuncImpManagementApp/FuncImpArbCalc/BrkFuncImpArbCalc/BrkFuncImpArbCalc/AEBpartFuncImpArbCalc ==
  AEBpartModStsArb : Inport
  AEBpartFuncImpAborCond : Inport  Port=2
  Compare To Constant : simulink/Logic and Bit Operations/Compare To Constant  relop=~=  const=ModSts.ActiveState  OutDataTypeStr=boolean
  Compare To Constant1 : simulink/Logic and Bit Operations/Compare To Constant  relop===  const=FALSE  OutDataTypeStr=boolean
  Constant : Constant  Value=ModSts.StandbyState
  Switch : Switch  Criteria=u2 ~= 0
  Switch1 : Switch  Criteria=u2 ~= 0
  AEBpartFuncImpArb : Outport
  wires:
  Switch/1 -> AEBpartFuncImpArb/1
  Switch1/1 -> Switch/3
  Constant/1 -> Switch1/3
  Compare To Constant1/1 -> Switch1/2
  AEBpartFuncImpAborCond/1 -> Compare To Constant1/1
  Compare To Constant/1 -> Switch/2
  AEBpartModStsArb/1 -> Compare To Constant/1, Switch/1, Switch1/1

== system TosAeb/FcwAebJa_Step_sys/ALG_AEBSystem/ALG_AEBController/AEB_FuncImpManagementApp/FuncImpArbCalc/BrkFuncImpArbCalc/BrkFuncImpArbCalc/EBAFuncImpArbCalc ==
  EBAModStsArb : Inport
  EBAFuncImpAborCond : Inport  Port=2
  Compare To Constant : simulink/Logic and Bit Operations/Compare To Constant  relop=~=  const=ModSts.ActiveState  OutDataTypeStr=boolean
  Compare To Constant1 : simulink/Logic and Bit Operations/Compare To Constant  relop===  const=FALSE  OutDataTypeStr=boolean
  Constant : Constant  Value=ModSts.StandbyState
  Switch : Switch  Criteria=u2 ~= 0
  Switch1 : Switch  Criteria=u2 ~= 0
  EBAFuncImpArb : Outport
  wires:
  EBAModStsArb/1 -> Switch1/1, Switch/1, Compare To Constant/1
  Compare To Constant/1 -> Switch/2
  EBAFuncImpAborCond/1 -> Compare To Constant1/1
  Compare To Constant1/1 -> Switch1/2
  Constant/1 -> Switch1/3
  Switch1/1 -> Switch/3
  Switch/1 -> EBAFuncImpArb/1

== system TosAeb/FcwAebJa_Step_sys/ALG_AEBSystem/ALG_AEBController/AEB_FuncImpManagementApp/FuncImpArbCalc/BrkFuncImpArbCalc/BrkFuncImpArbCalc/FCTBFuncImpArbCalc ==
  FCTBModStsArb : Inport
  FCTBFuncImpAborCond : Inport  Port=2
  Compare To Constant : simulink/Logic and Bit Operations/Compare To Constant  relop=~=  const=ModSts.ActiveState  OutDataTypeStr=boolean
  Compare To Constant1 : simulink/Logic and Bit Operations/Compare To Constant  relop===  const=FALSE  OutDataTypeStr=boolean
  Constant : Constant  Value=ModSts.StandbyState
  Switch : Switch  Criteria=u2 ~= 0
  Switch1 : Switch  Criteria=u2 ~= 0
  FCTBFuncImpArb : Outport
  wires:
  Switch/1 -> FCTBFuncImpArb/1
  Switch1/1 -> Switch/3
  Constant/1 -> Switch1/3
  Compare To Constant1/1 -> Switch1/2
  FCTBFuncImpAborCond/1 -> Compare To Constant1/1
  Compare To Constant/1 -> Switch/2
  FCTBModStsArb/1 -> Compare To Constant/1, Switch/1, Switch1/1

== system TosAeb/FcwAebJa_Step_sys/ALG_AEBSystem/ALG_AEBController/AEB_FuncImpManagementApp/FuncImpArbCalc/BrkFuncImpArbCalc/BrkFuncImpArbCalc/JA_PTW_AEBFuncImpArbCalc ==
  JA_PTW_AEBModStsArb : Inport
  JA_PTW_AEBFuncImpAborCond : Inport  Port=2
  Compare To Constant : simulink/Logic and Bit Operations/Compare To Constant  relop=~=  const=ModSts.ActiveState  OutDataTypeStr=boolean
  Compare To Constant1 : simulink/Logic and Bit Operations/Compare To Constant  relop===  const=FALSE  OutDataTypeStr=boolean
  Constant : Constant  Value=ModSts.StandbyState
  Switch : Switch  Criteria=u2 ~= 0
  Switch1 : Switch  Criteria=u2 ~= 0
  JA_PTW_AEBFuncImpArb : Outport
  wires:
  Switch/1 -> JA_PTW_AEBFuncImpArb/1
  Switch1/1 -> Switch/3
  Constant/1 -> Switch1/3
  Compare To Constant1/1 -> Switch1/2
  JA_PTW_AEBFuncImpAborCond/1 -> Compare To Constant1/1
  Compare To Constant/1 -> Switch/2
  JA_PTW_AEBModStsArb/1 -> Compare To Constant/1, Switch/1, Switch1/1

== system TosAeb/FcwAebJa_Step_sys/ALG_AEBSystem/ALG_AEBController/AEB_FuncImpManagementApp/FuncImpArbCalc/BrkFuncImpArbCalc/BrkFuncImpArbCalc/JA_cycAEBFuncImpArbCalc ==
  JA_cycAEBModStsArb : Inport
  JA_cycAEBFuncImpAborCond : Inport  Port=2
  Compare To Constant : simulink/Logic and Bit Operations/Compare To Constant  relop=~=  const=ModSts.ActiveState  OutDataTypeStr=boolean
  Compare To Constant1 : simulink/Logic and Bit Operations/Compare To Constant  relop===  const=FALSE  OutDataTypeStr=boolean
  Constant : Constant  Value=ModSts.StandbyState
  Switch : Switch  Criteria=u2 ~= 0
  Switch1 : Switch  Criteria=u2 ~= 0
  JA_cycAEBFuncImpArb : Outport
  wires:
  JA_cycAEBModStsArb/1 -> Switch1/1, Switch/1, Compare To Constant/1
  Compare To Constant/1 -> Switch/2
  JA_cycAEBFuncImpAborCond/1 -> Compare To Constant1/1
  Compare To Constant1/1 -> Switch1/2
  Constant/1 -> Switch1/3
  Switch1/1 -> Switch/3
  Switch/1 -> JA_cycAEBFuncImpArb/1

== system TosAeb/FcwAebJa_Step_sys/ALG_AEBSystem/ALG_AEBController/AEB_FuncImpManagementApp/FuncImpArbCalc/BrkFuncImpArbCalc/BrkFuncImpArbCalc/JA_pedAEBFuncImpArbCalc3 ==
  JA_pedAEBModStsArb : Inport
  JA_pedAEBFuncImpAborCond : Inport  Port=2
  Compare To Constant : simulink/Logic and Bit Operations/Compare To Constant  relop=~=  const=ModSts.ActiveState  OutDataTypeStr=boolean
  Compare To Constant1 : simulink/Logic and Bit Operations/Compare To Constant  relop===  const=FALSE  OutDataTypeStr=boolean
  Constant : Constant  Value=ModSts.StandbyState
  Switch : Switch  Criteria=u2 ~= 0
  Switch1 : Switch  Criteria=u2 ~= 0
  JA_pedAEBFuncImpArb : Outport
  wires:
  Switch/1 -> JA_pedAEBFuncImpArb/1
  Switch1/1 -> Switch/3
  Constant/1 -> Switch1/3
  Compare To Constant1/1 -> Switch1/2
  JA_pedAEBFuncImpAborCond/1 -> Compare To Constant1/1
  Compare To Constant/1 -> Switch/2
  JA_pedAEBModStsArb/1 -> Compare To Constant/1, Switch/1, Switch1/1

== system TosAeb/FcwAebJa_Step_sys/ALG_AEBSystem/ALG_AEBController/AEB_FuncImpManagementApp/FuncImpArbCalc/BrkFuncImpArbCalc/BrkFuncImpArbCalc/JA_vehAEBfullFuncImpArbCalc ==
  JA_vehAEBfullfullModStsArb : Inport
  JA_vehAEBfullFuncImpAborCond : Inport  Port=2
  Compare To Constant : simulink/Logic and Bit Operations/Compare To Constant  relop=~=  const=ModSts.ActiveState  OutDataTypeStr=boolean
  Compare To Constant1 : simulink/Logic and Bit Operations/Compare To Constant  relop===  const=FALSE  OutDataTypeStr=boolean
  Constant : Constant  Value=ModSts.StandbyState
  Switch : Switch  Criteria=u2 ~= 0
  Switch1 : Switch  Criteria=u2 ~= 0
  JA_vehAEBfullFuncImpArb : Outport
  wires:
  JA_vehAEBfullfullModStsArb/1 -> Switch1/1, Switch/1, Compare To Constant/1
  Compare To Constant/1 -> Switch/2
  JA_vehAEBfullFuncImpAborCond/1 -> Compare To Constant1/1
  Compare To Constant1/1 -> Switch1/2
  Constant/1 -> Switch1/3
  Switch1/1 -> Switch/3
  Switch/1 -> JA_vehAEBfullFuncImpArb/1

== system TosAeb/FcwAebJa_Step_sys/ALG_AEBSystem/ALG_AEBController/AEB_FuncImpManagementApp/FuncImpArbCalc/BrkFuncImpArbCalc/BrkFuncImpArbCalc/cycAEBfullFuncImpArbCalc ==
  cycAEBfullModStsArb : Inport
  cycAEBfullFuncImpAborCond : Inport  Port=2
  Compare To Constant : simulink/Logic and Bit Operations/Compare To Constant  relop=~=  const=ModSts.ActiveState  OutDataTypeStr=boolean
  Compare To Constant1 : simulink/Logic and Bit Operations/Compare To Constant  relop===  const=FALSE  OutDataTypeStr=boolean
  Constant : Constant  Value=ModSts.StandbyState
  Switch : Switch  Criteria=u2 ~= 0
  Switch1 : Switch  Criteria=u2 ~= 0
  cycAEBfullFuncImpArb : Outport
  wires:
  Switch/1 -> cycAEBfullFuncImpArb/1
  Switch1/1 -> Switch/3
  Constant/1 -> Switch1/3
  Compare To Constant1/1 -> Switch1/2
  cycAEBfullFuncImpAborCond/1 -> Compare To Constant1/1
  Compare To Constant/1 -> Switch/2
  cycAEBfullModStsArb/1 -> Compare To Constant/1, Switch/1, Switch1/1

== system TosAeb/FcwAebJa_Step_sys/ALG_AEBSystem/ALG_AEBController/AEB_FuncImpManagementApp/FuncImpArbCalc/BrkFuncImpArbCalc/BrkFuncImpArbCalc/cycAEBpartFuncImpArbCalc ==
  cycAEBpartModStsArb : Inport
  cycAEBpartFuncImpAborCond : Inport  Port=2
  Compare To Constant : simulink/Logic and Bit Operations/Compare To Constant  relop=~=  const=ModSts.ActiveState  OutDataTypeStr=boolean
  Compare To Constant1 : simulink/Logic and Bit Operations/Compare To Constant  relop===  const=FALSE  OutDataTypeStr=boolean
  Constant : Constant  Value=ModSts.StandbyState
  Switch : Switch  Criteria=u2 ~= 0
  Switch1 : Switch  Criteria=u2 ~= 0
  cycAEBpartFuncImpArb : Outport
  wires:
  cycAEBpartModStsArb/1 -> Switch1/1, Switch/1, Compare To Constant/1
  Compare To Constant/1 -> Switch/2
  cycAEBpartFuncImpAborCond/1 -> Compare To Constant1/1
  Compare To Constant1/1 -> Switch1/2
  Constant/1 -> Switch1/3
  Switch1/1 -> Switch/3
  Switch/1 -> cycAEBpartFuncImpArb/1

== system TosAeb/FcwAebJa_Step_sys/ALG_AEBSystem/ALG_AEBController/AEB_FuncImpManagementApp/FuncImpArbCalc/BrkFuncImpArbCalc/BrkFuncImpArbCalc/pedAEBfullFuncImpArbCalc ==
  pedAEBfullModStsArb : Inport
  pedAEBfullFuncImpAborCond : Inport  Port=2
  Compare To Constant : simulink/Logic and Bit Operations/Compare To Constant  relop=~=  const=ModSts.ActiveState  OutDataTypeStr=boolean
  Compare To Constant1 : simulink/Logic and Bit Operations/Compare To Constant  relop===  const=FALSE  OutDataTypeStr=boolean
  Constant : Constant  Value=ModSts.StandbyState
  Switch : Switch  Criteria=u2 ~= 0
  Switch1 : Switch  Criteria=u2 ~= 0
  pedAEBfullFuncImpArb : Outport
  wires:
  pedAEBfullModStsArb/1 -> Switch1/1, Switch/1, Compare To Constant/1
  Compare To Constant/1 -> Switch/2
  pedAEBfullFuncImpAborCond/1 -> Compare To Constant1/1
  Compare To Constant1/1 -> Switch1/2
  Constant/1 -> Switch1/3
  Switch1/1 -> Switch/3
  Switch/1 -> pedAEBfullFuncImpArb/1

== system TosAeb/FcwAebJa_Step_sys/ALG_AEBSystem/ALG_AEBController/AEB_FuncImpManagementApp/FuncImpArbCalc/BrkFuncImpArbCalc/BrkFuncImpSuppCondCalc/AEBfullFuncImpSuppCondCalc ==
  pedAEBfullModStsArb : Inport
  cycAEBfullModStsArb : Inport  Port=2
  EBAModStsArb : Inport  Port=3
  JA_PTW_AEBfullModStsArb : Inport  Port=4
  JA_cycAEBfullModStsArb : Inport  Port=5
  JA_pedAEBfullModStsArb : Inport  Port=6
  Compare To Constant{3..5,23..25} (6 alike) : simulink/Logic and Bit Operations/Compare To Constant  relop===  const=ModSts.ActiveState  OutDataTypeStr=boolean
  Logical Operator4 : Logic  Operator=OR  Inputs=6  AllPortsSameDT=off  OutDataTypeStr=boolean
  AEBfullFuncImpSuppCond : Outport
  wires:
  Compare To Constant3/1 -> Logical Operator4/4
  Compare To Constant4/1 -> Logical Operator4/5
  Compare To Constant5/1 -> Logical Operator4/6
  JA_pedAEBfullModStsArb/1 -> Compare To Constant5/1
  JA_cycAEBfullModStsArb/1 -> Compare To Constant4/1
  JA_PTW_AEBfullModStsArb/1 -> Compare To Constant3/1
  EBAModStsArb/1 -> Compare To Constant25/1
  Compare To Constant24/1 -> Logical Operator4/2
  Compare To Constant23/1 -> Logical Operator4/1
  cycAEBfullModStsArb/1 -> Compare To Constant24/1
  pedAEBfullModStsArb/1 -> Compare To Constant23/1
  Logical Operator4/1 -> AEBfullFuncImpSuppCond/1
  Compare To Constant25/1 -> Logical Operator4/3
